simulink model "AcasXuClosedLoop_m_1_deriv"  (Simulink 9.2)
settings: Solver=VariableStepAuto  StartTime=0.0  StopTime=25  RelTol=1e-3
libraries: neural x1560, simulink x1
(part 60 of 60)

== system AcasXuClosedLoop_m_1_deriv/AcasXu NN Controller/AcasXu_5_1/Layer 7/Delays 1 ==
  "p{7}" : Inport  PortDimensions=50
      mask Display: e = 0.06;c1 = [120 230 180]/255;c2 = ([120 230 180]+0)/(256+128);c3 = ([120 230 180]+128)/(256+128);patch([0 1 1 0],[0 0 1 1],c1);patch([0 1 1 1-e 1-e 0+e],[0 0 1 1-e 0+e 0+e],c2);patch([0 0 1 1-e 0+e 0+e],[0 1 1 1-e 1-e 0+e],c3);plot([0 1 1 0 0],[0 0 1 1 0]);
  mux : Mux  Inputs=1
      mask Display: e = 0.06;c1 = [120 150 230]/255;c2 = ([120 150 230]+0)/383;c3 = ([120 150 230]+128)/383;patch([0 1 1 0],[0 0 1 1],c1);patch([0 1 1 1-e 1-e 0+e],[0 0 1 1-e 0+e 0+e],c2);patch([0 0 1 1-e 0+e 0+e],[0 1 1 1-e 1-e 0+e],c3);plot([0 1 1 0 0],[0 0 1 1 0]);disp('Mux')
  "pd{7,6}" : Outport  VectorParamsAs1DForOutWhenUnconnected=on
      mask Display: e = 0.06;c1 = [120 230 180]/255;c2 = ([120 230 180]+0)/(256+128);c3 = ([120 230 180]+128)/(256+128);patch([0 1 1 0],[0 0 1 1],c1);patch([0 1 1 1-e 1-e 0+e],[0 0 1 1-e 0+e 0+e],c2);patch([0 0 1 1-e 0+e 0+e],[0 1 1 1-e 1-e 0+e],c3);plot([0 1 1 0 0],[0 0 1 1 0]);
  wires:
  mux/1 -> "pd{7,6}"/1
  "p{7}"/1 -> mux/1

== system AcasXuClosedLoop_m_1_deriv/AcasXu NN Controller/AcasXu_5_1/Layer 7/LW{7,6} ==
  "ad{7,6}" : Inport  PortDimensions=50
      mask Display: e = 0.06;c1 = [120 230 180]/255;c2 = ([120 230 180]+0)/(256+128);c3 = ([120 230 180]+128)/(256+128);patch([0 1 1 0],[0 0 1 1],c1);patch([0 1 1 1-e 1-e 0+e],[0 0 1 1-e 0+e 0+e],c2);patch([0 0 1 1-e 0+e 0+e],[0 1 1 1-e 1-e 0+e],c3);plot([0 1 1 0 0],[0 0 1 1 0]);
  "IW{7,6}(1,:)'" : Constant  Value=[0.129155999999999993033128475872217677533626556396484375;0.05413980000000000181170634050431544892489910125732421875;0.0190858999999999993935073661077694850973784923553466796875;-0.04048469999999999846540532644212362356483936309814453125;0.052825900000000002021405265395515016280114650726318359375;0.003863179999999999912618786623852429329417645931243896484375;0.0087121999999999998054445171646875678561627864837646484375;0.0118078999999999996461941265124551136977970600128173828125;0.01325759999999999959319207931685014045797288417816162109375;-0.0141925000000000002542410726391608477570116519927978515625;0.022441400000000000236610731008113361895084381103515625;-0.01004020000000000086004536825612376560457050800323486328125;-0.0229736000000000002707611912455831770785152912139892578125;0.004074580000000000389037690950999603956006467342376708984375;0.021553400000000000336086714014527387917041778564453125;0.0286048999999999990773158486945249023847281932830810546875;0.074522699999999997277910779303056187927722930908203125;-0.0133431000000000000993427562434590072371065616607666015625;0.024570000000000001560973572622970095835626125335693359375;0.03428269999999999928519400782533921301364898681640625;0.05175380000000000257909249512522364966571331024169921875;-0.0216865000000000009150458168960540206171572208404541015625;-0.0257152999999999999636290937132798717357218265533447265625;-0.00751723999999999968613106915427124476991593837738037109375;0.0206267000000000012838174967555460170842707157135009765625;0.043266699999999998216271279716238495893776416778564453125;0.030344900000000001039079933207176509313285350799560546875;-0.01262319999999999935169636700038608978502452373504638671875;0.091610899999999995113597606177791021764278411865234375;-0.0177129000000000001724398401847793138585984706878662109375;0.04911290000000000099955599353052093647420406341552734375;-0.0088655699999999997007460450504368054680526256561279296875;0.0032001799999999999517263926662735684658400714397430419921875;0.0173254000000000012160494833324264618568122386932373046875;-0.14455200000000001381295078317634761333465576171875;-0.043683899999999997731947587453760206699371337890625;0.002684230000000000122828414106379568693228065967559814453125;0.032446999999999996677768621111681568436324596405029296875;0.0152429000000000001990851927757830708287656307220458984375;-0.0198137000000000000621280804580237600021064281463623046875;-0.0043336599999999995957988829786700080148875713348388671875;-0.0400478999999999973891107174495118670165538787841796875;0.033016600000000000114663833983286167494952678680419921875;0.0022202900000000001974609364907564668101258575916290283203125;0.0529586999999999974431119653672794811427593231201171875;0.14690700000000000979838432613178156316280364990234375;0.03961789999999999756230550929103628732264041900634765625;0.0239576999999999984414245091102202422916889190673828125;-0.0272790000000000011637357744120890856720507144927978515625;0.002209599999999999932198679886141690076328814029693603515625]
      mask Display: e = 0.06;c1 = [120 150 230]/255;c2 = ([120 150 230]+0)/383;c3 = ([120 150 230]+128)/383;patch([0 1 1 0],[0 0 1 1],c1);patch([0 1 1 1-e 1-e 0+e],[0 0 1 1-e 0+e 0+e],c2);patch([0 0 1 1-e 0+e 0+e],[0 1 1 1-e 1-e 0+e],c3);plot([0 1 1 0 0],[0 0 1 1 0]);disp('weights');
  "IW{7,6}(2,:)'" : Constant  Value=[0.1016309999999999991171506508180755190551280975341796875;0.05803149999999999975042186406426480971276760101318359375;0.0165158000000000006191047674519722932018339633941650390625;-0.015820000000000000728306304154102690517902374267578125;0.056356999999999997374988680576279875822365283966064453125;-0.01251329999999999977033926512604011804796755313873291015625;0.0221403000000000016456169760203920304775238037109375;-0.0028751800000000001834854490567749962792731821537017822265625;-0.0257552999999999983338216935635500703938305377960205078125;-0.0210886999999999984189980040127920801751315593719482421875;-0.0005596929999999999960635932438890449702739715576171875;-0.0169908999999999997532196260863202041946351528167724609375;-0.036725399999999998323918504183893674053251743316650390625;0.0276125000000000016264767310758543317206203937530517578125;0.03080789999999999917434934104676358401775360107421875;0.03523980000000000167847957754929666407406330108642578125;0.007215230000000000427917701273372586001642048358917236328125;-0.00152334999999999992116583857892919695586897432804107666015625;0.07592190000000000049684700798025005497038364410400390625;0.03381320000000000158824065010776394046843051910400390625;0.050890999999999998737454376396271982230246067047119140625;-0.025947800000000000031352698215414420701563358306884765625;-0.0217855999999999987160048675605139578692615032196044921875;-0.0062254700000000003090594447030525770969688892364501953125;0.0171630000000000011717293801893902127631008625030517578125;0.0089971200000000008112355232015033834613859653472900390625;0.034409399999999999708588660496388911269605159759521484375;-0.0246867999999999983284926230453493189997971057891845703125;-0.000204352999999999999924948923535339417867362499237060546875;-0.01790529999999999899440439321551821194589138031005859375;0.0274702000000000001733724275254644453525543212890625;0.039373999999999999277466855573948123492300510406494140625;-0.004647179999999999887305701662398860207758843898773193359375;0.0298834000000000009344969242874867632053792476654052734375;0.0113077000000000003898659173273699707351624965667724609375;-0.040208399999999998308819471048991545103490352630615234375;0.01387490000000000077429174183407667442224919795989990234375;0.00449954999999999973037123623953448259271681308746337890625;0.05585790000000000199431582359466119669377803802490234375;-0.022018900000000000971578373309966991655528545379638671875;0.0156577000000000000901057006785777048207819461822509765625;-0.025918799999999998784350196956438594497740268707275390625;0.0315076999999999995960564547203830443322658538818359375;-0.00186027999999999996312560757161236324463970959186553955078125;0.0744831999999999994077626297439564950764179229736328125;0.0024199999999999998422095526251496266922913491725921630859375;0.039295900000000001661693005416964297182857990264892578125;-0.01215749999999999990396570836992395925335586071014404296875;-0.0241700000000000005118128143521971651352941989898681640625;-0.005932179999999999571447251156541824457235634326934814453125]
      mask Display: e = 0.06;c1 = [120 150 230]/255;c2 = ([120 150 230]+0)/383;c3 = ([120 150 230]+128)/383;patch([0 1 1 0],[0 0 1 1],c1);patch([0 1 1 1-e 1-e 0+e],[0 0 1 1-e 0+e 0+e],c2);patch([0 0 1 1-e 0+e 0+e],[0 1 1 1-e 1-e 0+e],c3);plot([0 1 1 0 0],[0 0 1 1 0]);disp('weights');
  "IW{7,6}(3,:)'" : Constant  Value=[0.10933199999999999862954069840270676650106906890869140625;0.0630319000000000018157919612349360249936580657958984375;0.02101039999999999852153820256717153824865818023681640625;-0.00816507999999999990736743171737543889321386814117431640625;0.0537926999999999988499865821722778491675853729248046875;-0.001960739999999999878810275077967162360437214374542236328125;-0.021033799999999998331379202909374726004898548126220703125;0.02076429999999999942428274835037882439792156219482421875;-0.03883240000000000302637914728620671667158603668212890625;0.034998599999999997767208270715855178423225879669189453125;-0.016451000000000000345057316053498652763664722442626953125;-0.01253970000000000084572349123845924623310565948486328125;0.00307172000000000000596855898038484156131744384765625;0.00672991000000000040570657944272170425392687320709228515625;-0.0132122999999999997389199535291481879539787769317626953125;0.02986969999999999902939862295170314610004425048828125;-0.00042358000000000000685729251159727937192656099796295166015625;-0.038547800000000000120170540185426943935453891754150390625;0.09546469999999999955786478267327765934169292449951171875;0.047177799999999998903366105196255375631153583526611328125;0.051149199999999998833555281407825532369315624237060546875;-0.0176094000000000007466471885209102765657007694244384765625;-0.026906800000000001549249617482928442768752574920654296875;-0.021035999999999999143796003409079276025295257568359375;0.004155709999999999959552354766856296919286251068115234375;0.005969809999999999873543377049145419732667505741119384765625;0.0069306899999999997674660079383102129213511943817138671875;0.002356529999999999909487957694409487885423004627227783203125;0.005828120000000000001938449400995523319579660892486572265625;-0.0238804999999999989501731079144519753754138946533203125;0.014721800000000000163691282750733080320060253143310546875;-0.0063441699999999996706367966226025600917637348175048828125;0.00138239000000000005104194844562925936770625412464141845703125;0.04358879999999999699245023521143593825399875640869140625;0.0291059000000000005214939591269285301677882671356201171875;-0.037611600000000001975397623255048529244959354400634765625;-0.0022017500000000001479649736069177379249595105648040771484375;0.002008300000000000189170901165880422922782599925994873046875;0.06174849999999999783373283435139455832540988922119140625;-0.0234507000000000014050538510446131112985312938690185546875;-0.0064372600000000002040678737103007733821868896484375;-0.04721329999999999971205255633321939967572689056396484375;0.044285499999999998477218099424135289154946804046630859375;0.0300522000000000011343814776409999467432498931884765625;0.03622630000000000294324564720227499492466449737548828125;-0.0031052599999999998779676158022766685462556779384613037109375;0.04081089999999999717861243198058218695223331451416015625;-0.032970300000000000995026283590050297789275646209716796875;-0.02833650000000000057642779438538127578794956207275390625;-0.001994590000000000078184125840152773889712989330291748046875]
      mask Display: e = 0.06;c1 = [120 150 230]/255;c2 = ([120 150 230]+0)/383;c3 = ([120 150 230]+128)/383;patch([0 1 1 0],[0 0 1 1],c1);patch([0 1 1 1-e 1-e 0+e],[0 0 1 1-e 0+e 0+e],c2);patch([0 0 1 1-e 0+e 0+e],[0 1 1 1-e 1-e 0+e],c3);plot([0 1 1 0 0],[0 0 1 1 0]);disp('weights');
  "IW{7,6}(4,:)'" : Constant  Value=[0.0802226000000000050160764430984272621572017669677734375;0.06659220000000000416751078091692761518061161041259765625;0.0151962999999999993916421914263992221094667911529541015625;-0.0027188099999999998900024333892133654444478452205657958984375;0.0526710999999999984755305604267050512135028839111328125;0.032754499999999998893773778263494023121893405914306640625;0.026544800000000000339550609851357876323163509368896484375;-0.028156699999999999783906190486959530971944332122802734375;0.034311500000000001719957509749292512424290180206298828125;-0.035046500000000001262545623603728017769753932952880859375;-0.0302761999999999996402433311004642746411263942718505859375;-0.03333529999999999826609808906141552142798900604248046875;-0.042901500000000002132960474909850745461881160736083984375;0.04314099999999999879296552762752980925142765045166015625;0.048135299999999998921129673590257880277931690216064453125;0.038769900000000002970867996054948889650404453277587890625;0.004112300000000000156641366544363336288370192050933837890625;0.0218882000000000000172750702631674357689917087554931640625;0.0777338999999999946677320394883281551301479339599609375;0.03511859999999999981667997417389415204524993896484375;0.035986999999999998156585689912390080280601978302001953125;-0.031796499999999998375965759578321012668311595916748046875;-0.0175277999999999996305621863257329096086323261260986328125;0.00826387999999999948996798337930158595554530620574951171875;0.034632099999999998996003114370978437364101409912109375;0.000556689999999999984327259117122821407974697649478912353515625;0.00972445000000000080164763716084053157828748226165771484375;-0.038076800000000000923083831594340153969824314117431640625;0.00016973199999999999377196802097245154072879813611507415771484375;-0.01560120000000000083095752501094466424547135829925537109375;0.034138700000000000989164306020029471255838871002197265625;0.048939299999999998080912888553939410485327243804931640625;0.00146587999999999991744548122341029738890938460826873779296875;0.031931700000000000361222163292040931992232799530029296875;-0.000652951000000000030508429116338220410398207604885101318359375;-0.023773400000000000087396756498492322862148284912109375;0.01653659999999999852260401667081168852746486663818359375;-0.0140570999999999994567456695904184016399085521697998046875;0.061569499999999999229061131700291298329830169677734375;-0.01562110000000000060993432526856850017793476581573486328125;0.039857099999999999473399014959795749746263027191162109375;-0.0035340699999999998330568740101398361730389297008514404296875;0.01230290000000000029400926138123395503498613834381103515625;-0.03450219999999999676010276061788317747414112091064453125;0.10059400000000000285904633301470312289893627166748046875;0.005854169999999999686457474723511040792800486087799072265625;0.042205499999999999960476060323344427160918712615966796875;0.0028430299999999998628030795089216553606092929840087890625;-0.01696430000000000160031987661568564362823963165283203125;-0.03907789999999999874802369959070347249507904052734375]
      mask Display: e = 0.06;c1 = [120 150 230]/255;c2 = ([120 150 230]+0)/383;c3 = ([120 150 230]+128)/383;patch([0 1 1 0],[0 0 1 1],c1);patch([0 1 1 1-e 1-e 0+e],[0 0 1 1-e 0+e 0+e],c2);patch([0 0 1 1-e 0+e 0+e],[0 1 1 1-e 1-e 0+e],c3);plot([0 1 1 0 0],[0 0 1 1 0]);disp('weights');
  "IW{7,6}(5,:)'" : Constant  Value=[0.0644234999999999946584949839234468527138233184814453125;0.0676617999999999941707073958241380751132965087890625;0.053453199999999999325606125921694911085069179534912109375;-0.0085532200000000002670930143722216598689556121826171875;0.04351069999999999937667638505445211194455623626708984375;-0.032311899999999997568078669019087101332843303680419921875;-0.033094199999999997230393233849099487997591495513916015625;0.045900900000000001421884832097930484451353549957275390625;0.01087879999999999923454563344193957163952291011810302734375;0.0305113000000000016143086867259626160375773906707763671875;0.0287780000000000014959145033799359225668013095855712890625;0.006749840000000000006519229600598919205367565155029296875;-0.0025054399999999998373267917628481882275082170963287353515625;0.0048527099999999996626787179820894380100071430206298828125;-0.03466940000000000299262836733760195784270763397216796875;0.005189209999999999656239424439263530075550079345703125;-0.0029356799999999999735422751001578944851644337177276611328125;-0.06833210000000000672315536576206795871257781982421875;0.05942150000000000209521289207259542308747768402099609375;0.04679640000000000188418169955184566788375377655029296875;0.053867699999999997528821182868341566063463687896728515625;0.02121939999999999937330130705959163606166839599609375;-0.0220021999999999995356603221807745285332202911376953125;0.016175900000000000000799360577730112709105014801025390625;-0.03153129999999999821813645439760875888168811798095703125;-0.006769260000000000034037217488958049216307699680328369140625;0.003733150000000000183486559279799621435813605785369873046875;0.01238170000000000069151351311802500276826322078704833984375;-0.0083628000000000000946354106190483435057103633880615234375;-0.021158799999999998442401505371890380047261714935302734375;0.015234799999999999731148392356772092171013355255126953125;0.00595681999999999982342568927151660318486392498016357421875;-0.038554900000000003057465391975711099803447723388671875;0.0192856999999999993711252699313263292424380779266357421875;0.00694677999999999962188468316526268608868122100830078125;0.004719860000000000201103578234551605419255793094635009765625;-0.0441790999999999989267251976343686692416667938232421875;-0.01189229999999999974613640318921170546673238277435302734375;0.07160329999999999472937162181551684625446796417236328125;-0.0253501999999999998169908366207891958765685558319091796875;-0.025249000000000000609734485124135972000658512115478515625;-0.00168049999999999991918964159509641831391490995883941650390625;0.034677699999999998803357215138021274469792842864990234375;0.044117700000000002746869398606577306054532527923583984375;-0.043318000000000002336353333021179423667490482330322265625;0.00949905000000000020177193249537594965659081935882568359375;0.041708399999999999641087100599179393611848354339599609375;5.68978000000000026597189728416736897997907362878322601318359375e-05;-0.029290599999999999969446662362315692007541656494140625;0.048275300000000000155697676973431953229010105133056640625]
      mask Display: e = 0.06;c1 = [120 150 230]/255;c2 = ([120 150 230]+0)/383;c3 = ([120 150 230]+128)/383;patch([0 1 1 0],[0 0 1 1],c1);patch([0 1 1 1-e 1-e 0+e],[0 0 1 1-e 0+e 0+e],c2);patch([0 0 1 1-e 0+e 0+e],[0 1 1 1-e 1-e 0+e],c3);plot([0 1 1 0 0],[0 0 1 1 0]);disp('weights');
  Mux : Mux  Inputs=5
      mask Display: e = 0.06;c1 = [120 150 230]/255;c2 = ([120 150 230]+0)/383;c3 = ([120 150 230]+128)/383;patch([0 1 1 0],[0 0 1 1],c1);patch([0 1 1 1-e 1-e 0+e],[0 0 1 1-e 0+e 0+e],c2);patch([0 0 1 1-e 0+e 0+e],[0 1 1 1-e 1-e 0+e],c3);plot([0 1 1 0 0],[0 0 1 1 0]);disp('Mux');
  dotprod{1..5} (5 alike) : neural/Weight Functions/dotprod
      settings: SourceProductName=Deep Learning Toolbox
  "lz{7,6}" : Outport  VectorParamsAs1DForOutWhenUnconnected=on
      mask Display: e = 0.06;c1 = [120 230 180]/255;c2 = ([120 230 180]+0)/(256+128);c3 = ([120 230 180]+128)/(256+128);patch([0 1 1 0],[0 0 1 1],c1);patch([0 1 1 1-e 1-e 0+e],[0 0 1 1-e 0+e 0+e],c2);patch([0 0 1 1-e 0+e 0+e],[0 1 1 1-e 1-e 0+e],c3);plot([0 1 1 0 0],[0 0 1 1 0]);
  wires:
  Mux/1 -> "lz{7,6}"/1
  dotprod5/1 -> Mux/5
  "IW{7,6}(5,:)'"/1 -> dotprod5/1
  dotprod4/1 -> Mux/4
  "IW{7,6}(4,:)'"/1 -> dotprod4/1
  dotprod3/1 -> Mux/3
  "IW{7,6}(3,:)'"/1 -> dotprod3/1
  dotprod2/1 -> Mux/2
  "IW{7,6}(2,:)'"/1 -> dotprod2/1
  dotprod1/1 -> Mux/1
  "ad{7,6}"/1 -> dotprod5/2, dotprod4/2, dotprod3/2, dotprod2/2, dotprod1/2
  "IW{7,6}(1,:)'"/1 -> dotprod1/1

== matlab function range1 ==
  data: u input double; y output double
function y = fcn(u)
if u > pi
    %m = floor(u/pi);
    y = -2*pi + u;
elseif u < -pi
    %m = ceil(u/pi);
    y = 2*pi + u;
else
    y = u;
end


end

== matlab function Argmin_Advisory_Output ==
  data: u input double; turn_rate output double; idx output double
function [turn_rate,idx] = fcn(u)
    [~,idx] = min(u);

    if idx == 1
        turn_rate = 0;
    elseif idx == 2
        turn_rate = deg2rad(1.5);
    elseif idx == 3
        turn_rate = deg2rad(-1.5);
    elseif idx == 4
        turn_rate = deg2rad(3.0);
    else
        turn_rate = deg2rad(-3.0);
    end
end

== stateflow chart DubinsPlant Ownship ==
  settings: updateMethod=CONTINUOUS
  state DubinDynamics
    du:
    %ownship
    x1_dot = 807*cos(x3);
    x2_dot = 807*sin(x3);
    x3_dot = u;
    % intruder
    x4_dot = 807*cos(x6);
    x5_dot = 807*sin(x6);
    x6_dot = 0;
    % nn inputs
    x7_dot = - ((-x4+x1)*(807*cos(x6)))/sqrt((x4-x1)^2+(x2-x5)^2) + ((-x4+x1)*(807*cos(x3)))/sqrt((x4-x1)^2+(x2-x5)^2) + ((x2-x5)*(807*sin(x3)))/sqrt((x4-x1)^2+(x2-x5)^2) - ((x2-x5)*(807*sin(x6)))/sqrt((x4-x1)^2+(x2-x5)^2);
    x8_dot = ((x4-x1)*(x4-x1+sqrt(((x4-x1)^2)+(x5-x2)^2)*(807*sin(x6))))/(sqrt((x4-x1)^2+(x5-x2)^2)*((x4-x1)^2+(x5-x2)^2+x4*sqrt(x5^2+x4^2-2*x4*x1+x1^2+x2^2-2*x5*x2)-x1*sqrt(x5^2+x4^2-2*x4*x1+x1^2+x2^2-2*x5*x2))) - (2*(1+(x4-x1)/(sqrt((x4-x1)^2+(x5-x2)^2)))*(x5-x2)*(807*cos(x6)))/(eps+((x4-x1+sqrt((x4-x1)^2+(x5-x2)^2))^2*(1+(x5-x2^2)/(x4-x1+sqrt((x4-x1)^2+(x5-x2)^2))^2))) - (2*(-1-(x4-x1)/(sqrt((x4-x1)^2+(x5-x2)^2)))*(x5-x2)*(807*cos(x3)))/(eps+((x4-x1+sqrt((x4-x1)^2+(x5-x2)^2))^2*(1+(x5-x2^2)/(x4-x1+sqrt((x4-x1)^2+(x5-x2)^2))^2))) - ((x4-x1)*(x4-x1+sqrt(((x4-x1)^2)+(x5-x2)^2)*(807*sin(x3))))/(sqrt((x4-x1)^2+(x5-x2)^2)*((x4-x1)^2+(x5-x2)^2+ x4*sqrt(x5^2+x4^2-2*x4*x1+x1^2+x2^2-2*x5*x2)-x1*sqrt(x5^2+x4^2-2*x4*x1+x1^2+x2^2-2*x5*x2)))  ;
    x9_dot = 0 - u;
    % OUTPUTS
    dist = x7;%
    theta = x8;
    heading_diff = x9;
    prev_adv = idx;
    %other outputs (visualization)
    x = x1;
    y = x2;
    heading = x3;
  (default) -> DubinDynamics : {x1 = 0;
      | x2 = 0;
      | x3 = 1.5708;
      | x4 = 0;
      | x5 = 25000;
      | x6 = -1.5708;
      | x7 = 25000;
      | x8 = 0;
      | x9 = 0;}
  data: u input double; dist output double; theta output double; heading_diff output double; x1 local double; x2 local double; x3 local double; idx input double; x4 local double; x5 local double; x6 local double; x output double; y output double; heading output double; prev_adv output double; x7 local double; x8 local double; x9 local double
  data x1 settings: updateMethod=SF_CONTINUOUS_TIME_DATA
  data x2 settings: updateMethod=SF_CONTINUOUS_TIME_DATA
  data x3 settings: updateMethod=SF_CONTINUOUS_TIME_DATA
  data x4 settings: updateMethod=SF_CONTINUOUS_TIME_DATA
  data x5 settings: updateMethod=SF_CONTINUOUS_TIME_DATA
  data x6 settings: updateMethod=SF_CONTINUOUS_TIME_DATA
  data x7 settings: updateMethod=SF_CONTINUOUS_TIME_DATA
  data x8 settings: updateMethod=SF_CONTINUOUS_TIME_DATA
  data x9 settings: updateMethod=SF_CONTINUOUS_TIME_DATA

== matlab function range ==
  data: u input double; y output double
function y = fcn(u)
if u > pi
    %m = floor(u/pi);
    y = -2*pi + u;
elseif u < -pi
    %m = ceil(u/pi);
    y = 2*pi + u;
else
    y = u;
end


end
